simulink model "SdbRxSigProc"  (R2022b) (continued)
== system SdbRxSigProc/SdbRxSigProc_Runnable_sys == (continued)
      settings: EnsureOutportIsVirtual=on
  OGMCmprSpdFbNgcc_OGMCmprSpdFbNgcc_write : Outport  Port=113
      settings: EnsureOutportIsVirtual=on
  OGMFlowFbNgcc_OGMFlowFbNgcc_write : Outport  Port=114
      settings: EnsureOutportIsVirtual=on
  OGMHwVersNgcc_OGMHwVersNgcc_write : Outport  Port=115
      settings: EnsureOutportIsVirtual=on
  OGMIntErrNgcc_OGMIntErrNgcc_write : Outport  Port=116
      settings: EnsureOutportIsVirtual=on
  OGMOvrVoltRangErrNgcc_OGMOvrVoltRangErrNgcc_write : Outport  Port=117
      settings: EnsureOutportIsVirtual=on
  OGMRunTiNgcc_OGMRunTiNgcc_write : Outport  Port=118
      settings: EnsureOutportIsVirtual=on
  OGMSwVersNgcc_OGMSwVersNgcc_write : Outport  Port=119
      settings: EnsureOutportIsVirtual=on
  OGMTempErrNgcc_OGMTempErrNgcc_write : Outport  Port=120
      settings: EnsureOutportIsVirtual=on
  OGMUndVoltRangErrNgcc_OGMUndVoltRangErrNgcc_write : Outport  Port=121
      settings: EnsureOutportIsVirtual=on
  OxygenConcFbFrmOGMNgcc_OxygenConcFbFrmOGMNgcc_write : Outport  Port=122
      settings: EnsureOutportIsVirtual=on
  OxygenConcFltNgcc_OxygenConcFltNgcc_write : Outport  Port=123
      settings: EnsureOutportIsVirtual=on
  OxygenConcSnsrElecErrNgcc_OxygenConcSnsrElecErrNgcc_write : Outport  Port=124
      settings: EnsureOutportIsVirtual=on
  OxygenConcSnsrIntErrNgcc_OxygenConcSnsrIntErrNgcc_write : Outport  Port=125
      settings: EnsureOutportIsVirtual=on
  OxygenConcSnsrOvrVoltRangErrNgcc_OxygenConcSnsrOvrVoltRangErrNgcc_write : Outport  Port=126
      settings: EnsureOutportIsVirtual=on
  OxygenConcSnsrStsFrmOGMNgcc_OxygenConcSnsrStsFrmOGMNgcc_write : Outport  Port=127
      settings: EnsureOutportIsVirtual=on
  OxygenConcSnsrStsNgcc_OxygenConcSnsrStsNgcc_write : Outport  Port=128
      settings: EnsureOutportIsVirtual=on
  OxygenConcSnsrTempErrNgcc_OxygenConcSnsrTempErrNgcc_write : Outport  Port=129
      settings: EnsureOutportIsVirtual=on
  OxygenConcSnsrUndVoltRangErrNgcc_OxygenConcSnsrUndVoltRangErrNgcc_write : Outport  Port=130
      settings: EnsureOutportIsVirtual=on
  OxygenGenrErrInfoNgcc_OxygenGenrErrInfoNgcc_write : Outport  Port=131
      settings: EnsureOutportIsVirtual=on
  OxygenSplyAvlStsNgcc_OxygenSplyAvlStsNgcc_write : Outport  Port=132
      settings: EnsureOutportIsVirtual=on
  OxygenSplyErrNgcc_OxygenSplyErrNgcc_write : Outport  Port=133
      settings: EnsureOutportIsVirtual=on
  OxygenTubeBlkNgcc_OxygenTubeBlkNgcc_write : Outport  Port=134
      settings: EnsureOutportIsVirtual=on
  PassSeatPosPercNgcc_PassSeatPosPercNgcc_write : Outport  Port=135
      settings: EnsureOutportIsVirtual=on
  PassSeatSts2_Ngcc_PassSeatSts2_write : Outport  Port=136
      settings: EnsureOutportIsVirtual=on
  PassSeatVentnLvlSts2Ngcc_PassSeatVentnLvlSts2Ngcc_write : Outport  Port=137
      settings: EnsureOutportIsVirtual=on
  PhaseCurrentFbNgcc_PhaseCurrentFbNgcc_write : Outport  Port=138
      settings: EnsureOutportIsVirtual=on
  PosnBriefNgcc_PosnBriefNgcc_write : Outport  Port=139
      settings: EnsureOutportIsVirtual=on
  PressureFbNgcc_PressureFbNgcc_write : Outport  Port=140
      settings: EnsureOutportIsVirtual=on
  PressureFltNgcc_PressureFltNgcc_write : Outport  Port=141
      settings: EnsureOutportIsVirtual=on
  PressureSnsrErrNgcc_PressureSnsrErrNgcc_write : Outport  Port=142
      settings: EnsureOutportIsVirtual=on
  PwrCnsForHvacHeatrElecRe_PwrCnsForHvacHeatrElecRe_write : Outport  Port=143
      settings: EnsureOutportIsVirtual=on
  RefrLpSwStsNgcc_RefrLpSwStsNgcc_write : Outport  Port=144
      settings: EnsureOutportIsVirtual=on
  RefrtorVlvEnaNgcc_RefrtorVlvEnaNgcc_write : Outport  Port=145
      settings: EnsureOutportIsVirtual=on
  RoadInclnNgcc_RoadInclnNgcc_write : Outport  Port=146
      settings: EnsureOutportIsVirtual=on
  RuranACModeSetNgcc_RuranACModeSetNgcc_write : Outport  Port=147
      settings: EnsureOutportIsVirtual=on
  SceneModSeld_Ngcc_SceneModSeld_write : Outport  Port=148
      settings: EnsureOutportIsVirtual=on
  SeatBackAngleRowFirstDrvrNgcc_SeatBackAngleRowFirstDrvrNgcc_write : Outport  Port=149
      settings: EnsureOutportIsVirtual=on
  SeatBackAngleRowFirstPassNgcc_SeatBackAngleRowFirstPassNgcc_write : Outport  Port=150
      settings: EnsureOutportIsVirtual=on
  SeatOccptAtRowSecDrSide2_Ngcc_SeatOccptAtRowSecDrSide2_write : Outport  Port=151
      settings: EnsureOutportIsVirtual=on
  SeatOccptAtRowSecMid2_Ngcc_SeatOccptAtRowSecMid2_write : Outport  Port=152
      settings: EnsureOutportIsVirtual=on
  SeatOccptAtRowSecPasSide2_Ngcc_SeatOccptAtRowSecPasSide2_write : Outport  Port=153
      settings: EnsureOutportIsVirtual=on
  SeatOccptAtRowThrdLe2Ngcc_SeatOccptAtRowThrdLe2Ngcc_write : Outport  Port=154
      settings: EnsureOutportIsVirtual=on
  SeatOccptAtRowThrdRi2Ngcc_SeatOccptAtRowThrdRi2Ngcc_write : Outport  Port=155
      settings: EnsureOutportIsVirtual=on
  SportModeThmgtRequestNgcc_SportModeThmgtRequestNgcc_write : Outport  Port=156
      settings: EnsureOutportIsVirtual=on
  TCSCooltFlowNgcc_TCSCooltFlowNgcc_write : Outport  Port=157
      settings: EnsureOutportIsVirtual=on
  TCSCooltTNgcc_TCSCooltTNgcc_write : Outport  Port=158
      settings: EnsureOutportIsVirtual=on
  TcsDoorEleBailReqNgcc_TcsDoorEleBailReqNgcc_write : Outport  Port=159
      settings: EnsureOutportIsVirtual=on
  TcsFanReqNgcc_TcsFanReqNgcc_write : Outport  Port=160
      settings: EnsureOutportIsVirtual=on
  TcsModSwtReqNgcc_TcsModSwtReqNgcc_write : Outport  Port=161
      settings: EnsureOutportIsVirtual=on
  TcsOvrheatdProtnStsNgcc_TcsOvrheatdProtnStsNgcc_write : Outport  Port=162
      settings: EnsureOutportIsVirtual=on
  TcsSemiconReqNgcc_TcsSemiconReqNgcc_write : Outport  Port=163
      settings: EnsureOutportIsVirtual=on
  TcsTmrOverReqNgcc_TcsTmrOverReqNgcc_write : Outport  Port=164
      settings: EnsureOutportIsVirtual=on
  TcsTNowCtrlReqNgcc_TcsTNowCtrlReqNgcc_write : Outport  Port=165
      settings: EnsureOutportIsVirtual=on
  TcsTopDoorEleBailReqNgcc_TcsTopDoorEleBailReqNgcc_write : Outport  Port=166
      settings: EnsureOutportIsVirtual=on
  TelmOxygenValReqNgcc_TelmOxygenValReqNgcc_write : Outport  Port=167
      settings: EnsureOutportIsVirtual=on
  TelmThermoTankECOSetNgcc_TelmThermoTankECOSetNgcc_write : Outport  Port=168
      settings: EnsureOutportIsVirtual=on
  TelmThermoTankTNgcc_TelmThermoTankTNgcc_write : Outport  Port=169
      settings: EnsureOutportIsVirtual=on
  ThermHvacRecircReqEnaNgcc_ThermHvacRecircReqEnaNgcc_write : Outport  Port=170
      settings: EnsureOutportIsVirtual=on
  ThermHvacRecircReqNgcc_ThermHvacRecircReqNgcc_write : Outport  Port=171
      settings: EnsureOutportIsVirtual=on
  ThrdRowSeatHeatgActlPwrCnsLeNgcc_ThrdRowSeatHeatgActlPwrCnsLeNgcc_write : Outport  Port=172
      settings: EnsureOutportIsVirtual=on
  ThrdRowSeatHeatgActlPwrCnsRiNgcc_ThrdRowSeatHeatgActlPwrCnsRiNgcc_write : Outport  Port=173
      settings: EnsureOutportIsVirtual=on
  ThrdRowSeatHeatgDesPwrCnsLeNgcc_ThrdRowSeatHeatgDesPwrCnsLeNgcc_write : Outport  Port=174
      settings: EnsureOutportIsVirtual=on
  ThrdRowSeatHeatgDesPwrCnsRiNgcc_ThrdRowSeatHeatgDesPwrCnsRiNgcc_write : Outport  Port=175
      settings: EnsureOutportIsVirtual=on
  ThrdRowSeatVentnActlPwrCnsLeNgcc_ThrdRowSeatVentnActlPwrCnsLeNgcc_write : Outport  Port=176
      settings: EnsureOutportIsVirtual=on
  ThrdRowSeatVentnActlPwrCnsRiNgcc_ThrdRowSeatVentnActlPwrCnsRiNgcc_write : Outport  Port=177
      settings: EnsureOutportIsVirtual=on
  ThrdRowSeatVentnDesPwrCnsLeNgcc_ThrdRowSeatVentnDesPwrCnsLeNgcc_write : Outport  Port=178
      settings: EnsureOutportIsVirtual=on
  ThrdRowSeatVentnDesPwrCnsRiNgcc_ThrdRowSeatVentnDesPwrCnsRiNgcc_write : Outport  Port=179
      settings: EnsureOutportIsVirtual=on
  UseEvapReqTMinNewNgcc_UseEvapReqTMinNewNgcc_write : Outport  Port=180
      settings: EnsureOutportIsVirtual=on
  VehTiAndDataNgcc_VehTiAndDataNgcc_write : Outport  Port=181
      settings: EnsureOutportIsVirtual=on
  VlvVoltFltNgcc_VlvVoltFltNgcc_write : Outport  Port=182
      settings: EnsureOutportIsVirtual=on
  WarnLvlMsgNgcc_WarnLvlMsgNgcc_write : Outport  Port=183
      settings: EnsureOutportIsVirtual=on
  WasRespLevelNgcc_WasRespLevelNgcc_write : Outport  Port=184
      settings: EnsureOutportIsVirtual=on
  WaterDepthInformationNgcc_WaterDepthInformationNgcc_write : Outport  Port=185
      settings: EnsureOutportIsVirtual=on
  WeatherQualityAQINgcc_WeatherQualityAQINgcc_write : Outport  Port=186
      settings: EnsureOutportIsVirtual=on
  WinPosnStsDrv_WinPosnStsAtDrv_write : Outport  Port=187
      settings: EnsureOutportIsVirtual=on
  ActvnOfWshrFrntSafe_Ngcc_ActvnOfWshrFrntSafe_write : Outport  Port=188
      settings: EnsureOutportIsVirtual=on
  AirMtrlSnsrInAirQlyIdx_AirMtrlSnsrInAirQlyIdx_write : Outport  Port=189
      settings: EnsureOutportIsVirtual=on
  AirTAftHeatElmEstimd_AirTAftHeatElmEstimd_write : Outport  Port=190
      settings: EnsureOutportIsVirtual=on
  AirTFromHeatgEstimd_Ngcc_AirTFromHeatgEstimd_write : Outport  Port=191
      settings: EnsureOutportIsVirtual=on
  AmbTOutOfVeh_AmbTOutOfVeh_write : Outport  Port=192
      settings: EnsureOutportIsVirtual=on
  AmbTRaw_Ngcc_AmbTRaw_write : Outport  Port=193
      settings: EnsureOutportIsVirtual=on
  AsyFanSts_AsyFanSts_write : Outport  Port=194
      settings: EnsureOutportIsVirtual=on
  BltLockStAtDrvr_Ngcc_BltLockStAtDrvr_write : Outport  Port=195
      settings: EnsureOutportIsVirtual=on
  BltLockStAtPass_Ngcc_BltLockStAtPass_write : Outport  Port=196
      settings: EnsureOutportIsVirtual=on
  BltLockStAtRowSecLe_Ngcc_BltLockStAtRowSecLe_write : Outport  Port=197
      settings: EnsureOutportIsVirtual=on
  BltLockStAtRowSecMid_Ngcc_BltLockStAtRowSecMid_write : Outport  Port=198
      settings: EnsureOutportIsVirtual=on
  BltLockStAtRowSecRi_Ngcc_BltLockStAtRowSecRi_write : Outport  Port=199
      settings: EnsureOutportIsVirtual=on
  BltLockStAtRowThrdLe_Ngcc_BltLockStAtRowThrdLe_write : Outport  Port=200
      settings: EnsureOutportIsVirtual=on
  BltLockStAtRowThrdRi_Ngcc_BltLockStAtRowThrdRi_write : Outport  Port=201
      settings: EnsureOutportIsVirtual=on
  CamHeatrActv_CamHeatrActv_write : Outport  Port=202
      settings: EnsureOutportIsVirtual=on
  CarTiGlb_Ngcc_CarTiGlb_write : Outport  Port=203
      settings: EnsureOutportIsVirtual=on
  ClimaCmd_Ngcc_ClimaCmd_write : Outport  Port=204
      settings: EnsureOutportIsVirtual=on
  ClimaPwrAllwd_Ngcc_ClimaPwrAllwd_write : Outport  Port=205
      settings: EnsureOutportIsVirtual=on
  ClimaRqrd1ConnGen2_ClimaRqrd1ConnGen2_write : Outport  Port=206
      settings: EnsureOutportIsVirtual=on
  ClimaRqrdFromRem1_ClimaRqrdFromRem1_write : Outport  Port=207
      settings: EnsureOutportIsVirtual=on
  ClimaTmr_ClimaTmr_write : Outport  Port=208
      settings: EnsureOutportIsVirtual=on
  ClimaTmrStsRqrd_ClimaTmrStsRqrd_write : Outport  Port=209
      settings: EnsureOutportIsVirtual=on
  ClngRqrdFromHmi_ClngRqrdFromHmi_write : Outport  Port=210
      settings: EnsureOutportIsVirtual=on
  ClngRqrdFromTelm_ClngRqrdFromTelm_write : Outport  Port=211
      settings: EnsureOutportIsVirtual=on
  ClngRqrdFromTelmConnGen2_ClngRqrdFromTelmConnGen2_write : Outport  Port=212
      settings: EnsureOutportIsVirtual=on
  CmpmtInAirPmAQI_Ngcc_CmpmtInAirPmAQI_write : Outport  Port=213
      settings: EnsureOutportIsVirtual=on
  CmpmtInAirPmDnsty_Ngcc_CmpmtInAirPmDnsty_write : Outport  Port=214
      settings: EnsureOutportIsVirtual=on
  CmptmtClngPreStrtReqNgcc_CmptmtClngPreStrtReq_write : Outport  Port=215
      settings: EnsureOutportIsVirtual=on
  CmptmtCoolgSts_Ngcc_CmptmtCoolgSts_write : Outport  Port=216
      settings: EnsureOutportIsVirtual=on
  CmptmtRelHum_Ngcc_CmptmtRelHum_write : Outport  Port=217
      settings: EnsureOutportIsVirtual=on
  CoolgLoad_CoolgLoad_write : Outport  Port=218
      settings: EnsureOutportIsVirtual=on
  CoolgReqForDispCen_CoolgReqForDispCen_write : Outport  Port=219
      settings: EnsureOutportIsVirtual=on
  CoolgReqForDrvrDisp_CoolgReqForDrvrDisp_write : Outport  Port=220
      settings: EnsureOutportIsVirtual=on
  CooltHeatrAvlStsForPrkgClima_CooltHeatrAvlStsForPrkgClima_write : Outport  Port=221
      settings: EnsureOutportIsVirtual=on
  CooltTForPrkgClima_CooltTForPrkgClima_write : Outport  Port=222
      settings: EnsureOutportIsVirtual=on
  CooltTInHeatElm_CooltTInHeatElm_write : Outport  Port=223
      settings: EnsureOutportIsVirtual=on
  CooltVlvClsd_CooltVlvClsd_write : Outport  Port=224
      settings: EnsureOutportIsVirtual=on
  CupHoldrDesPwr_CupHoldrDesPwr_write : Outport  Port=225
      settings: EnsureOutportIsVirtual=on
  DefrstSts_Ngcc_DefrstSts_write : Outport  Port=226
      settings: EnsureOutportIsVirtual=on
  DoorDrvrReSts_DoorDrvrReSts_write : Outport  Port=227
      settings: EnsureOutportIsVirtual=on
  DoorDrvrSts_Ngcc_DoorDrvrSts_write : Outport  Port=228
      settings: EnsureOutportIsVirtual=on
  DoorPassReSts_DoorPassReSts_write : Outport  Port=229
      settings: EnsureOutportIsVirtual=on
  DoorPassSts_Ngcc_DoorPassSts_write : Outport  Port=230
      settings: EnsureOutportIsVirtual=on
  DrvModReq_Ngcc_DrvModReq_write : Outport  Port=231
      settings: EnsureOutportIsVirtual=on
  DrvrAirCdnrSetg_Ngcc_DrvrAirCdnrSetg_write : Outport  Port=232
      settings: EnsureOutportIsVirtual=on
  DrvrSeatHeatgActlPwrCns_Ngcc_DrvrSeatHeatgActlPwrCns_write : Outport  Port=233
      settings: EnsureOutportIsVirtual=on
  DrvrSeatHeatgDesPwrCns_Ngcc_DrvrSeatHeatgDesPwrCns_write : Outport  Port=234
      settings: EnsureOutportIsVirtual=on
  DrvrSeatVentnDesPwrCns_Ngcc_DrvrSeatVentnDesPwrCns_write : Outport  Port=235
      settings: EnsureOutportIsVirtual=on
  EcuKeepActvFromHeatg_EcuKeepActvFromHeatg_write : Outport  Port=236
      settings: EnsureOutportIsVirtual=on
  EgyCnsAllwdForClima_Ngcc_EgyCnsAllwdForClima_write : Outport  Port=237
      settings: EnsureOutportIsVirtual=on
  EgySave_EgySave_write : Outport  Port=238
      settings: EnsureOutportIsVirtual=on
  EngReqInHybForHeatg_EngReqInHybForHeatg_write : Outport  Port=239
      settings: EnsureOutportIsVirtual=on
  EngSt1WdSts_Ngcc_EngSt1WdSts_write : Outport  Port=240
      settings: EnsureOutportIsVirtual=on
  EngT_Ngcc_EngT_write : Outport  Port=241
      settings: EnsureOutportIsVirtual=on
  FogOutOfVeh_FogOutOfVeh_write : Outport  Port=242
      settings: EnsureOutportIsVirtual=on
  HeatdCupPwrCnsAct_HeatdCupPwrCnsAct_write : Outport  Port=243
      settings: EnsureOutportIsVirtual=on
  HeatgPwrDmdEstimd_HeatgPwrDmdEstimd_write : Outport  Port=244
      settings: EnsureOutportIsVirtual=on
  HeatrActvnReq_HeatrActvnReq_write : Outport  Port=245
      settings: EnsureOutportIsVirtual=on
  HMIClimaEgySaveReq_Ngcc_HMIClimaEgySaveReq_write : Outport  Port=246
      settings: EnsureOutportIsVirtual=on
  HmiClimaFrntAutReq_HmiClimaFrntAutReq_write : Outport  Port=247
      settings: EnsureOutportIsVirtual=on
  HmiClimaReAutReq_HmiClimaReAutReq_write : Outport  Port=248
      settings: EnsureOutportIsVirtual=on
  HmiCmptmtAirDistbnFrntLe_HmiCmptmtAirDistbnFrntLe_write : Outport  Port=249
      settings: EnsureOutportIsVirtual=on
  HmiCmptmtAirDistbnRe_HmiCmptmtAirDistbnRe_write : Outport  Port=250
      settings: EnsureOutportIsVirtual=on
  HmiCmptmtCoolgReq_HmiCmptmtCoolgReq_write : Outport  Port=251
      settings: EnsureOutportIsVirtual=on
  HmiDefrstMaxReq_HmiDefrstMaxReq_write : Outport  Port=252
      settings: EnsureOutportIsVirtual=on
  HmiHvacFanLvlFrnt_HmiHvacFanLvlFrnt_write : Outport  Port=253
      settings: EnsureOutportIsVirtual=on
  HmiHvacFanLvlRe_HmiHvacFanLvlRe_write : Outport  Port=254
      settings: EnsureOutportIsVirtual=on
  HmiMaxACReq_HmiMaxACReq_write : Outport  Port=255
      settings: EnsureOutportIsVirtual=on
  HmiPassTempSyncSet_HmiPassTempSyncSet_write : Outport  Port=256
      settings: EnsureOutportIsVirtual=on
  HpcDeiceReq_Ngcc_HpcDeiceReq_write : Outport  Port=257
      settings: EnsureOutportIsVirtual=on
  HumOutOfVeh_HumOutOfVeh_write : Outport  Port=258
      settings: EnsureOutportIsVirtual=on
  HvacFanIFrnt_HvacFanIFrnt_write : Outport  Port=259
      settings: EnsureOutportIsVirtual=on
  HvacFanIRe_HvacFanIRe_write : Outport  Port=260
      settings: EnsureOutportIsVirtual=on
  HvacFanMotURe_HvacFanMotURe_write : Outport  Port=261
      settings: EnsureOutportIsVirtual=on
  HvacFanSpdFrnt_HvacFanSpdFrnt_write : Outport  Port=262
      settings: EnsureOutportIsVirtual=on
  HvBattCoolgPwrHeatgMgrThermPred_HvBattCoolgPwrHeatgMgrThermPred_write : Outport  Port=263
      settings: EnsureOutportIsVirtual=on
  HvPwrEgyPrio_Ngcc_HvPwrEgyPrio_write : Outport  Port=264
      settings: EnsureOutportIsVirtual=on
  IHUAPSts_Ngcc_IHUAPSts_write : Outport  Port=265
      settings: EnsureOutportIsVirtual=on
  IncomingAirQlyCtrlFromHmi_Ngcc_IncomingAirQlyCtrlFromHmi_write : Outport  Port=266
      settings: EnsureOutportIsVirtual=on
  IncomingAirQlyPopUpReq_Ngcc_IncomingAirQlyPopUpReq_write : Outport  Port=267
      settings: EnsureOutportIsVirtual=on
  InPm25FanErr_Ngcc_InPm25FanErr_write : Outport  Port=268
      settings: EnsureOutportIsVirtual=on
  InPm25SnsrSts_Ngcc_InPm25SnsrSts_write : Outport  Port=269
      settings: EnsureOutportIsVirtual=on
  InteCleanUnpleSmell_Ngcc_InteCleanUnpleSmell_write : Outport  Port=270
      settings: EnsureOutportIsVirtual=on
  IsgMotTInt_IsgMotTInt_write : Outport  Port=271
      settings: EnsureOutportIsVirtual=on
  LoadForCmprForAirCdn_LoadForCmprForAirCdn_write : Outport  Port=272
      settings: EnsureOutportIsVirtual=on
  LockgCenSts_Ngcc_LockgCenSts_write : Outport  Port=273
      settings: EnsureOutportIsVirtual=on
  MemSaveFlgForCooltHeatrActvnCtrl_MemSaveFlgForCooltHeatrActvnCtrl_write : Outport  Port=274
      settings: EnsureOutportIsVirtual=on
  MemSaveFlgForCooltHeatrCtrl_MemSaveFlgForCooltHeatrCtrl_write : Outport  Port=275
      settings: EnsureOutportIsVirtual=on
  MemSaveFlgForFuTInHeatrMdl_MemSaveFlgForFuTInHeatrMdl_write : Outport  Port=276
      settings: EnsureOutportIsVirtual=on
  MemSaveFlgForHeatgInpMgr_MemSaveFlgForHeatgInpMgr_write : Outport  Port=277
      settings: EnsureOutportIsVirtual=on
  MemSaveFlgForSnsrMdlCooltT_MemSaveFlgForSnsrMdlCooltT_write : Outport  Port=278
      settings: EnsureOutportIsVirtual=on
  MemWrForCooltHeatrActvnCtrl_MemWrForCooltHeatrActvnCtrl_write : Outport  Port=279
      settings: EnsureOutportIsVirtual=on
  MemWrForCooltHeatrCtrl_MemWrForCooltHeatrCtrl_write : Outport  Port=280
      settings: EnsureOutportIsVirtual=on
  MemWrForFuTInFuHeatrMdl_MemWrForFuTInFuHeatrMdl_write : Outport  Port=281
      settings: EnsureOutportIsVirtual=on
  MemWrForHeatgInpMgr_MemWrForHeatgInpMgr_write : Outport  Port=282
      settings: EnsureOutportIsVirtual=on
  MemWrForSnsrMdlCooltT_MemWrForSnsrMdlCooltT_write : Outport  Port=283
      settings: EnsureOutportIsVirtual=on
  NetReqForHeatg_NetReqForHeatg_write : Outport  Port=284
      settings: EnsureOutportIsVirtual=on
  OnBdChrgrHndlSts_OnBdChrgrHndlSts_write : Outport  Port=285
      settings: EnsureOutportIsVirtual=on
  PassSeatHeatgActlPwrCns_Ngcc_PassSeatHeatgActlPwrCns_write : Outport  Port=286
      settings: EnsureOutportIsVirtual=on
  PassSeatHeatgDesPwrCns_Ngcc_PassSeatHeatgDesPwrCns_write : Outport  Port=287
      settings: EnsureOutportIsVirtual=on
  PassSeatVentnActlPwrCns_Ngcc_PassSeatVentnActlPwrCns_write : Outport  Port=288
      settings: EnsureOutportIsVirtual=on
  PassSeatVentnDesPwrCns_Ngcc_PassSeatVentnDesPwrCns_write : Outport  Port=289
      settings: EnsureOutportIsVirtual=on
  SplyUForClimaCtrl_SplyUForClimaCtrl_write : Outport  Port=290
      settings: EnsureOutportIsVirtual=on
  PostDrvgClimaReq_Ngcc_PostDrvgClimaReq_write : Outport  Port=291
      settings: EnsureOutportIsVirtual=on
  PrkgHeatgActv_PrkgHeatgActv_write : Outport  Port=292
      settings: EnsureOutportIsVirtual=on
  PrkgHeatrStrtd_PrkgHeatrStrtd_write : Outport  Port=293
      settings: EnsureOutportIsVirtual=on
  PwrCnsDesForSeatHeatg_PwrCnsDesForSeatHeatg_write : Outport  Port=294
      settings: EnsureOutportIsVirtual=on
  PwrCnsDesForSeatVent_PwrCnsDesForSeatVent_write : Outport  Port=295
      settings: EnsureOutportIsVirtual=on
  PwrCnsDurgEngStopForFuHeatr_PwrCnsDurgEngStopForFuHeatr_write : Outport  Port=296
      settings: EnsureOutportIsVirtual=on
  PwrCnsDurgEngStopForHvacCooltVlv_PwrCnsDurgEngStopForHvacCooltVlv_write : Outport  Port=297
      settings: EnsureOutportIsVirtual=on
  PwrCnsDurgEngStopForWtrPmpAux_PwrCnsDurgEngStopForWtrPmpAux_write : Outport  Port=298
      settings: EnsureOutportIsVirtual=on
  PwrCnsForFuHeatr_PwrCnsForFuHeatr_write : Outport  Port=299
      settings: EnsureOutportIsVirtual=on
  PwrCnsForHvacCooltVlv_PwrCnsForHvacCooltVlv_write : Outport  Port=300
      settings: EnsureOutportIsVirtual=on
  PwrCnsForHvacHeatrElecFrnt_PwrCnsForHvacHeatrElecFrnt_write : Outport  Port=301
      settings: EnsureOutportIsVirtual=on
  PwrCnsForSeatHeatg_PwrCnsForSeatHeatg_write : Outport  Port=302
      settings: EnsureOutportIsVirtual=on
  PwrCnsForWtrPmpAux_PwrCnsForWtrPmpAux_write : Outport  Port=303
      settings: EnsureOutportIsVirtual=on
  PwrStsForPrkgHeatrElec_PwrStsForPrkgHeatrElec_write : Outport  Port=304
      settings: EnsureOutportIsVirtual=on
  RainfallAmnt_Ngcc_RainfallAmnt_write : Outport  Port=305
      settings: EnsureOutportIsVirtual=on
  RemStrtHvCtrlReq_Ngcc_RemStrtHvCtrlReq_write : Outport  Port=306
      settings: EnsureOutportIsVirtual=on
  SeatHeatDurgClimaEnad_SeatHeatDurgClimaEnad_write : Outport  Port=307
      settings: EnsureOutportIsVirtual=on
  SeatHeatDurgClimaEnadConnGen2_SeatHeatDurgClimaEnadConnGen2_write : Outport  Port=308
      settings: EnsureOutportIsVirtual=on
  SeatHeatDurgClimaEnadFromTelm_Ngcc_SeatHeatDurgClimaEnadFromTelm_write : Outport  Port=309
      settings: EnsureOutportIsVirtual=on
  SecRowSeatHeatgActlPwrCnsLe_Ngcc_SecRowSeatHeatgActlPwrCnsLe_write : Outport  Port=310
      settings: EnsureOutportIsVirtual=on
  SecRowSeatHeatgActlPwrCnsRi_Ngcc_SecRowSeatHeatgActlPwrCnsRi_write : Outport  Port=311
      settings: EnsureOutportIsVirtual=on
  SecRowSeatHeatgDesPwrCnsLe_Ngcc_SecRowSeatHeatgDesPwrCnsLe_write : Outport  Port=312
      settings: EnsureOutportIsVirtual=on
  SecRowSeatHeatgDesPwrCnsRi_Ngcc_SecRowSeatHeatgDesPwrCnsRi_write : Outport  Port=313
      settings: EnsureOutportIsVirtual=on
  SecRowSeatVentnActlPwrCnsLe_Ngcc_SecRowSeatVentnActlPwrCnsLe_write : Outport  Port=314
      settings: EnsureOutportIsVirtual=on
  SecRowSeatVentnActlPwrCnsRi_Ngcc_SecRowSeatVentnActlPwrCnsRi_write : Outport  Port=315
      settings: EnsureOutportIsVirtual=on
  SecRowSeatVentnDesPwrCnsLe_Ngcc_SecRowSeatVentnDesPwrCnsLe_write : Outport  Port=316
      settings: EnsureOutportIsVirtual=on
  SecRowSeatVentnDesPwrCnsRi_Ngcc_SecRowSeatVentnDesPwrCnsRi_write : Outport  Port=317
      settings: EnsureOutportIsVirtual=on
  SessionActvOutOfVeh_SessionActvOutOfVeh_write : Outport  Port=318
      settings: EnsureOutportIsVirtual=on
  SteerWhlHeatgDesPwr_Ngcc_SteerWhlHeatgDesPwr_write : Outport  Port=319
      settings: EnsureOutportIsVirtual=on
  SteerWhlHeatgDurgClimaEnad_SteerWhlHeatgDurgClimaEnad_write : Outport  Port=320
      settings: EnsureOutportIsVirtual=on
  SteerWhlHeatgDurgClimEandFromTelmConnGen2_SteerWhlHeatgDurgClimEandFromTelmConnGen2_write : Outport  Port=321
      settings: EnsureOutportIsVirtual=on
  SteerWhlHeatgPwrAct_Ngcc_SteerWhlHeatgPwrAct_write : Outport  Port=322
      settings: EnsureOutportIsVirtual=on
  StrtRemSts_StrtRemSts1WdStrtRemSts_write : Outport  Port=323
      settings: EnsureOutportIsVirtual=on
  StrtRemSts1WdStrtRemSts_Ngcc_StrtRemSts1WdStrtRemSts_write : Outport  Port=324
      settings: EnsureOutportIsVirtual=on
  SunCurtPosnSts_Ngcc_SunCurtPosnSts_write : Outport  Port=325
      settings: EnsureOutportIsVirtual=on
  SunData_SunData_write : Outport  Port=326
      settings: EnsureOutportIsVirtual=on
  SunLoadOutOfVeh_SunLoadOutOfVeh_write : Outport  Port=327
      settings: EnsureOutportIsVirtual=on
  SunRoofPosnSts_Ngcc_SunRoofPosnSts_write : Outport  Port=328
      settings: EnsureOutportIsVirtual=on
  TelmPM25Req_Ngcc_TelmPM25Req_write : Outport  Port=329
      settings: EnsureOutportIsVirtual=on
  TiDrvgCycPred_TiDrvgCycPred_write : Outport  Port=330
      settings: EnsureOutportIsVirtual=on
  TrSts_Ngcc_TrSts_write : Outport  Port=331
      settings: EnsureOutportIsVirtual=on
  VehModMngtGlbSafe1_Ngcc_VehModMngtGlbSafe1_write : Outport  Port=332
      settings: EnsureOutportIsVirtual=on
  VehSpdLgt_Ngcc_VehSpdLgtSafe_write : Outport  Port=333
      settings: EnsureOutportIsVirtual=on
  WadeProtnSts_Ngcc_WadeProtnSts_write : Outport  Port=334
      settings: EnsureOutportIsVirtual=on
  WinGlbCmd_Ngcc_WinGlbCmd_write : Outport  Port=335
      settings: EnsureOutportIsVirtual=on
  WinPosnStsAtDrvrReNgcc_WinPosnStsAtDrvrReNgcc_write : Outport  Port=336
      settings: EnsureOutportIsVirtual=on
  WinPosnStsAtPassReNgcc_WinPosnStsAtPassRe_write : Outport  Port=337
      settings: EnsureOutportIsVirtual=on
  WinPosnStsPass_WinPosnStsAtPass_write : Outport  Port=338
      settings: EnsureOutportIsVirtual=on
  WipgInfo_Ngcc_WipgInfo_write : Outport  Port=339
      settings: EnsureOutportIsVirtual=on
  WtrPmpActvnReq_WtrPmpActvnReq_write : Outport  Port=340
      settings: EnsureOutportIsVirtual=on
  wires:
  ActvnOfWshrFrntSafe_ActvnOfWshrFrntSafe_read/1 -> Terminator_1/1
  AirTFromHeatgEstimd_AirTFromHeatgEstimd_read/1 -> Terminator_2/1
  AmbTRaw_AmbTRaw_read/1 -> Terminator_3/1
  BltLockStAtDrvr_BltLockStAtDrvr_read/1 -> Terminator_4/1
  BltLockStAtPass_BltLockStAtPass_read/1 -> Terminator_5/1
  BltLockStAtRowSecLe_BltLockStAtRowSecLe_read/1 -> Terminator_6/1
  BltLockStAtRowSecMid_BltLockStAtRowSecMid_read/1 -> Terminator_7/1
  BltLockStAtRowSecRi_BltLockStAtRowSecRi_read/1 -> Terminator_8/1
  BltLockStAtRowThrdLe_BltLockStAtRowThrdLe_read/1 -> Terminator_9/1
  BltLockStAtRowThrdRi_BltLockStAtRowThrdRi_read/1 -> Terminator_10/1
  BookTrvlClimaActvReq_BookTrvlClimaActvReq_read/1 -> Terminator_11/1
  CamSts_CamSts_read/1 -> Terminator_12/1
  CarTiGlb_CarTiGlb_read/1 -> Terminator_13/1
  ClimaCfgForSnsrHumPrsnt1_ClimaCfgForSnsrHumPrsnt1_read/1 -> Terminator_14/1
  ClimaCmd_ClimaCmd_read/1 -> Terminator_15/1
  ClimaPwrAllwd_ClimaPwrAllwd_read/1 -> Terminator_16/1
  CmpmtInAirPmAQI_CmpmtInAirPmAQI_read/1 -> Terminator_17/1
  CmpmtInAirPmDnsty_CmpmtInAirPmDnsty_read/1 -> Terminator_18/1
  CmptmtCoolgSts_CmptmtCoolgSts_read/1 -> Terminator_19/1
  CmptmtFrntWindDewT_CmptmtFrntWindDewT_read/1 -> Terminator_20/1
  CmptmtFrntWindT_CmptmtFrntWindT_read/1 -> Terminator_21/1
  CmptmtRelHum_CmptmtRelHum_read/1 -> Terminator_22/1
  CoolgLoadGroup_CoolgLoadGroup_read/1 -> Terminator_23/1
  DefrstSts_DefrstSts_read/1 -> Terminator_24/1
  DoorDrvrSts_DoorDrvrSts_read/1 -> Terminator_25/1
  DoorLeReSts_DoorLeReSts_read/1 -> Terminator_26/1
  DoorPassSts_DoorPassSts_read/1 -> Terminator_27/1
  DoorRiReSts_DoorRiReSts_read/1 -> Terminator_28/1
  DrvModReq_DrvModReq_read/1 -> Terminator_29/1
  DrvrAirCdnrSetg_DrvrAirCdnrSetg_read/1 -> Terminator_30/1
  DrvrSeatHeatgActlPwrCns_DrvrSeatHeatgActlPwrCns_read/1 -> Terminator_31/1
  DrvrSeatHeatgDesPwrCns_DrvrSeatHeatgDesPwrCns_read/1 -> Terminator_32/1
  DrvrSeatVentnActlPwrCns_DrvrSeatVentnActlPwrCns_read/1 -> Terminator_33/1
  DrvrSeatVentnDesPwrCns_DrvrSeatVentnDesPwrCns_read/1 -> Terminator_34/1
  EgyCnsAllwdForClima_EgyCnsAllwdForClima_read/1 -> Terminator_35/1
  EngT_EngT_read/1 -> Terminator_36/1
  HMIClimaEgySaveReq_HMIClimaEgySaveReq_read/1 -> Terminator_37/1
  HpcDeiceReq_HpcDeiceReq_read/1 -> Terminator_38/1
  HvacFanSts2HvacFanBattI2_HvacFanSts2HvacFanBattI2_read/1 -> Terminator_39/1
  HvacFanSts2HvacFanMotU2_HvacFanSts2HvacFanMotU2_read/1 -> Terminator_40/1
  HvacFanSts3HvacFanBattI_HvacFanSts3HvacFanBattI_read/1 -> Terminator_41/1
  HvacFanSts3HvacFanSpdFd_HvacFanSts3HvacFanSpdFd_read/1 -> Terminator_42/1
  HvPwrEgyPrio_HvPwrEgyPrio_read/1 -> Terminator_43/1
  IHUAPSts_IHUAPSts_read/1 -> Terminator_44/1
  IncomingAirQlyCtrlFromHmi_IncomingAirQlyCtrlFromHmi_read/1 -> Terminator_45/1
  IncomingAirQlyPopUpReq_IncomingAirQlyPopUpReq_read/1 -> Terminator_46/1
  InPm25FanErr_InPm25FanErr_read/1 -> Terminator_47/1
  InPm25SnsrElecErr_InPm25SnsrElecErr_read/1 -> Terminator_48/1
  InPm25SnsrSts_InPm25SnsrSts_read/1 -> Terminator_49/1
  InteCleanUnpleSmell_InteCleanUnpleSmell_read/1 -> Terminator_50/1
  IsgMotT_IsgMotT_read/1 -> Terminator_51/1
  LeSolarData_LeSolarData_read/1 -> Terminator_52/1
  LockgCenSts_LockgCenSts_read/1 -> Terminator_53/1
  OnBdChrgrHndlSts1_OnBdChrgrHndlSts1_read/1 -> Terminator_54/1
  PassSeatHeatgActlPwrCns_PassSeatHeatgActlPwrCns_read/1 -> Terminator_55/1
  PassSeatHeatgDesPwrCns_PassSeatHeatgDesPwrCns_read/1 -> Terminator_56/1
  PassSeatVentnActlPwrCns_PassSeatVentnActlPwrCns_read/1 -> Terminator_57/1
  PassSeatVentnDesPwrCns_PassSeatVentnDesPwrCns_read/1 -> Terminator_58/1
  PostDrvgClimaReq_PostDrvgClimaReq_read/1 -> Terminator_59/1
  RainfallAmnt_RainfallAmnt_read/1 -> Terminator_60/1
  RelHumSnsrQf_RelHumSnsrQf_read/1 -> Terminator_61/1
  RemStrtHvCtrlReq_RemStrtHvCtrlReq_read/1 -> Terminator_62/1
  RiSolarData_RiSolarData_read/1 -> Terminator_63/1
  SceneModSeld_SceneModSeld_read/1 -> Terminator_64/1
  SeatHeatDurgClimaEnadFromTelm_SeatHeatDurgClimaEnadFromTelm_read/1 -> Terminator_65/1
  SecRowSeatHeatgActlPwrCnsLe_SecRowSeatHeatgActlPwrCnsLe_read/1 -> Terminator_66/1
  SecRowSeatHeatgActlPwrCnsRi_SecRowSeatHeatgActlPwrCnsRi_read/1 -> Terminator_67/1
  SecRowSeatHeatgDesPwrCnsLe_SecRowSeatHeatgDesPwrCnsLe_read/1 -> Terminator_68/1
  SecRowSeatHeatgDesPwrCnsRi_SecRowSeatHeatgDesPwrCnsRi_read/1 -> Terminator_69/1
  SecRowSeatVentnActlPwrCnsLe_SecRowSeatVentnActlPwrCnsLe_read/1 -> Terminator_70/1
  SecRowSeatVentnActlPwrCnsRi_SecRowSeatVentnActlPwrCnsRi_read/1 -> Terminator_71/1
  SecRowSeatVentnDesPwrCnsLe_SecRowSeatVentnDesPwrCnsLe_read/1 -> Terminator_72/1
  SecRowSeatVentnDesPwrCnsRi_SecRowSeatVentnDesPwrCnsRi_read/1 -> Terminator_73/1
  SolarSnsrVluQf_SolarSnsrVluQf_read/1 -> Terminator_74/1
  SteerWhlHeatgDesPwr_SteerWhlHeatgDesPwr_read/1 -> Terminator_75/1
  SteerWhlHeatgPwrAct_SteerWhlHeatgPwrAct_read/1 -> Terminator_76/1
  StrtRemSts1WdStrtRemSts_StrtRemSts1WdStrtRemSts_read/1 -> Terminator_77/1
  SunCurtPosnSts_SunCurtPosnSts_read/1 -> Terminator_78/1
  SunRoofPosnSts_SunRoofPosnSts_read/1 -> Terminator_79/1
  TelmPM25Req_TelmPM25Req_read/1 -> Terminator_80/1
  TrSts_TrSts_read/1 -> Terminator_81/1
  VehBattU_VehBattU_read/1 -> Terminator_82/1
  VehSpdLgt_VehSpdLgt_read/1 -> Terminator_83/1
  VehTiAndData_VehTiAndData_read/1 -> Terminator_84/1
  WadeProtnSts_WadeProtnSts_read/1 -> Terminator_85/1
  WinGlbCmd_WinGlbCmd_read/1 -> Terminator_86/1
  WinPosnStsAtDrvr_WinPosnStsAtDrvr_read/1 -> Terminator_87/1
  WinPosnStsAtPass_WinPosnStsAtPass_read/1 -> Terminator_88/1
  WinPosnStsAtReLe_WinPosnStsAtReLe_read/1 -> Terminator_89/1
  WinPosnStsAtReRi_WinPosnStsAtReRi_read/1 -> Terminator_90/1
  WipgInfo_WipgInfo_read/1 -> Terminator_91/1
  VehModMngtGlbSafe1_VehModMngtGlbSafe1_read/1 -> Terminator_92/1
  VehModMngtGlbSafe1_VehModMngtGlbSafe1_IsUpdated_read/1 -> Terminator_93/1
  AACAirClngErrSts_AACAirClngErrSts_read/1 -> Terminator_94/1
  AACAirClngSts_AACAirClngSts_read/1 -> Terminator_95/1
  AFUFragraCh1AvlTiPrec_AFUFragraCh1AvlTiPrec_read/1 -> Terminator_96/1
  AFUFragraCh1AvlTiPrec_AFUFragraCh1AvlTiPrec_IsUpdated_read/1 -> Terminator_97/1
  AFUFragraCh2AvlTiPrec_AFUFragraCh2AvlTiPrec_read/1 -> Terminator_98/1
  AFUFragraCh2AvlTiPrec_AFUFragraCh2AvlTiPrec_IsUpdated_read/1 -> Terminator_99/1
  AFUFragraCh3AvlTiPrec_AFUFragraCh3AvlTiPrec_read/1 -> Terminator_100/1
  AFUFragraCh3AvlTiPrec_AFUFragraCh3AvlTiPrec_IsUpdated_read/1 -> Terminator_101/1
  AFUFragraCh4AvlTiPrec_AFUFragraCh4AvlTiPrec_read/1 -> Terminator_102/1
  AFUFragraCh4AvlTiPrec_AFUFragraCh4AvlTiPrec_IsUpdated_read/1 -> Terminator_103/1
  AFUFragraCh5AvlTiPrec_AFUFragraCh5AvlTiPrec_read/1 -> Terminator_104/1
  AFUFragraCh5AvlTiPrec_AFUFragraCh5AvlTiPrec_IsUpdated_read/1 -> Terminator_105/1
  AirFragCh1OvrdDy_AirFragCh1OvrdDy_read/1 -> Terminator_106/1
  AirFragCh2OvrdDy_AirFragCh2OvrdDy_read/1 -> Terminator_107/1
  AirFragCh3OvrdDy_AirFragCh3OvrdDy_read/1 -> Terminator_108/1
  AirFragCh4OvrdDy_AirFragCh4OvrdDy_read/1 -> Terminator_109/1
  AirFragCh5OvrdDy_AirFragCh5OvrdDy_read/1 -> Terminator_110/1
  AirPtcEnad_AirPtcEnad_read/1 -> Terminator_111/1
  AirPtcHVPwrActl_AirPtcHVPwrActl_read/1 -> Terminator_112/1
  AirPtcHVPwrActlFristLe_AirPtcHVPwrActlFristLe_read/1 -> Terminator_113/1
  AirPtcHVPwrActlFristRi_AirPtcHVPwrActlFristRi_read/1 -> Terminator_114/1
  AirPtcHVPwrActlSecLe_AirPtcHVPwrActlSecLe_read/1 -> Terminator_115/1
  AirPtcModeReq_AirPtcModeReq_read/1 -> Terminator_116/1
  AirSrcStsFb_AirSrcStsFb_read/1 -> Terminator_117/1
  AltitudeInfrom_AltitudeInfrom_read/1 -> Terminator_118/1
  AMBSts_AMBSts_read/1 -> Terminator_119/1
  BackflushVlvErr_BackflushVlvErr_read/1 -> Terminator_120/1
  BusCurrentFb_BusCurrentFb_read/1 -> Terminator_121/1
  ChgFilElmWarn_ChgFilElmWarn_read/1 -> Terminator_122/1
  ClimaCfgForDrvrSeatVentWthSmf2_ClimaCfgForDrvrSeatVentWthSmf2_read/1 -> Terminator_123/1
  ClimaCfgForPassSeatVentWthSmf2_ClimaCfgForPassSeatVentWthSmf2_read/1 -> Terminator_124/1
  CmptmtOxygenConcQF_CmptmtOxygenConcQF_read/1 -> Terminator_125/1
  CmptmtOxygenConcVlu_CmptmtOxygenConcVlu_read/1 -> Terminator_126/1
  CoolgFanErr_CoolgFanErr_read/1 -> Terminator_127/1
  CourseDirFromNav_CourseDirFromNav_read/1 -> Terminator_128/1
  CurrentCongestionInformation_CurrentCongestionInformation_read/1 -> Terminator_129/1
  CurrentTunnelInformation_CurrentTunnelInformation_read/1 -> Terminator_130/1
  DcDcActvd_DcDcActvd_read/1 -> Terminator_131/1
  DcDcPwrLim_DcDcPwrLim_read/1 -> Terminator_132/1
  DHUResdSig10_DHUResdSig10_read/1 -> Terminator_133/1
  DHUResdSig11_DHUResdSig11_read/1 -> Terminator_134/1
  DHUResdSig12_DHUResdSig12_read/1 -> Terminator_135/1
  DHUResdSig13_DHUResdSig13_read/1 -> Terminator_136/1
  DHUResdSig3_DHUResdSig3_read/1 -> Terminator_137/1
  DHUResdSig4_DHUResdSig4_read/1 -> Terminator_138/1
  DHUResdSig5_DHUResdSig5_read/1 -> Terminator_139/1
  DHUResdSig6_DHUResdSig6_read/1 -> Terminator_140/1
  DHUResdSig7_DHUResdSig7_read/1 -> Terminator_141/1
  DHUResdSig8_DHUResdSig8_read/1 -> Terminator_142/1
  DHUResdSig9_DHUResdSig9_read/1 -> Terminator_143/1
  DispHvBattLvlOfChrg_DispHvBattLvlOfChrg_read/1 -> Terminator_144/1
  DropTopPosnSts_DropTopPosnSts_read/1 -> Terminator_145/1
  DrvModReqType1ForCompile_DrvModReqType1ForCompile_read/1 -> Terminator_146/1
  DrvrSeatPosPerc_DrvrSeatPosPerc_read/1 -> Terminator_147/1
  DrvrSeatPosPerc_DrvrSeatPosPerc_IsUpdated_read/1 -> Terminator_148/1
  DrvrSeatSts_DrvrSeatSts_read/1 -> Terminator_149/1
  DrvrSeatVentnActlPwrCns2_DrvrSeatVentnActlPwrCns2_read/1 -> Terminator_150/1